simulink model "baopoco"  (Simulink 7.5)
settings: Solver=ode45  StartTime=0.0  StopTime=10.0  RelTol=1e-3
undefined symbols (not defined in the file or in its folder): quant
libraries: xbsIndex_r4 x4199, simulink x56, xps_library x32, dspsigops x24, casper_library_misc x9, casper_library_sources x4
(part 1 of 46)
settings Model: Creator=zaki  LastModifiedBy=zaki
settings DebuggingCC: StrictBusMsg=Warning

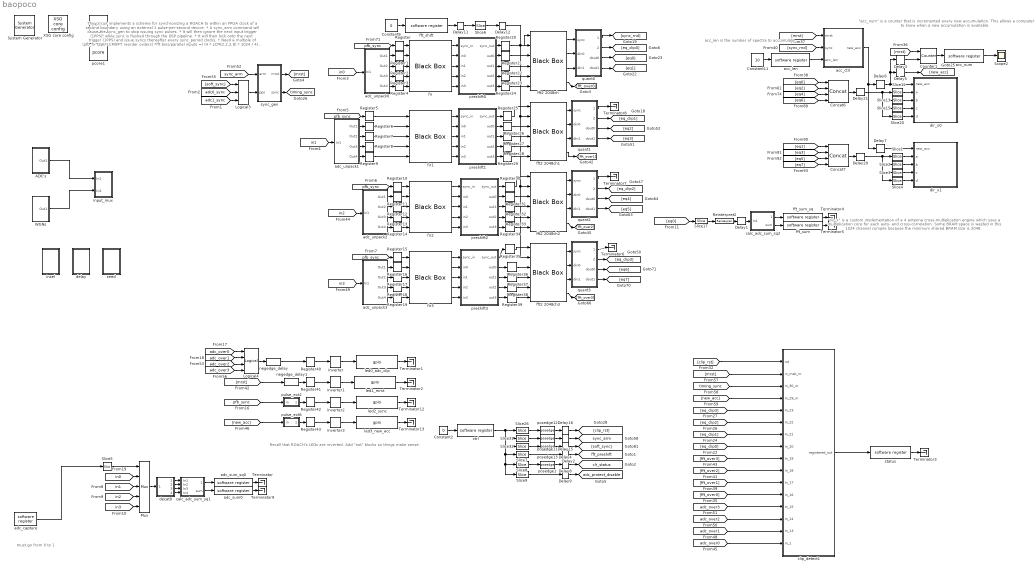
== system baopoco ==
  note: acc_len is the number of
      | spectra to accumulate.
  note: "acc_num" is a counter that is
      | incremented every new accumulation.
      | This allows a computer to know when
      | a new accumulation is available.
  note: "dir_x" is a custom implementation
      | of a 4 antenna cross-multiplication
      | engine which uses a multiplication
      | core for each auto- and cross-correlation.
      | Some BRAM space is wasted in this 1024
      | channel compile because the minimum shared
      | BRAM size is 2048.
  note: Recall that ROACH's LEDs are inverted.
      | Add "not" blocks so things make sense.
  note: This circuit implements a scheme for synchronizing a ROACH
      | to within an FPGA clock of a second boundary using an external 1 pulse-per-second device.
      |
      | * A sync_arm command will cause the sync_gen to stop issuing sync pulses.
      | * It will then ignore the next input trigger (1PPS), while sync is flushed through the DSP pipeline.
      | * It will then lock onto the next trigger (1PPS) and issue syncs thereafter every sync_period clocks.
      | * Need a multiple of (pfb_fir taps*LCM(FFT reorder orders) *fft bins/parallel inputs =)
      | (4 * LCM(2,2,2,8) * 1024 / 4) = 8192) for sync_period for simulation
      | * Trigger off either ADC's 1PPS inputs
  note: must go from 0 to 1
  " System Generator" : xbsIndex_r4/ System Generator  Tag=genX  SystemSampleTime=-1  xilinxfamily=virtex5  part=xc5vsx95t  speed=-1  package=ff1136  synthesis_tool=XST  clock_wrapper=Clock Enables  directory=./baopoco/sysgen  proj_type=Project Navigator  Synth_file=XST Defaults  Impl_file=ISE Defaults  testbench=off  simulink_period=1  sysclk_period=6.6667  dcm_input_clock_period=100  incr_netlist=off  trim_vbits=Everywhere in SubSystem  dbl_ovrd=According to Block Masks  core_generation=According to Block Masks  run_coregen=off  deprecated_control=off  eval_field=0  block_type=sysgen
      settings: block_version=11.4
  ADC's : Subsystem
  Concat6 : xbsIndex_r4/Concat  num_inputs=4  dbl_ovrd=off  block_type=concat
      settings: block_version=10.1.3
  Concat7 : xbsIndex_r4/Concat  num_inputs=4  dbl_ovrd=off  block_type=concat
      settings: block_version=10.1.3
  Constant11 : Constant  Value=10  OutDataType=sfix(16)  OutScaling=2^0
  Constant2 : Constant  Value=0  OutDataType=sfix(16)  OutScaling=2^0
  Constant9 : Constant  Value=0  OutDataType=sfix(16)  OutScaling=2^0
  Counter1 : xbsIndex_r4/Counter  cnt_type=Free Running  cnt_to=Inf  operation=Up  start_count=0  cnt_by_val=1  arith_type=Unsigned  n_bits=32  bin_pt=0  load_pin=off  rst=on  en=on  explicit_period=off  period=1  dbl_ovrd=off  use_behavioral_HDL=off  implementation=Fabric  use_rpm=on  block_type=counter
      settings: xl_area=[0, 0, 0, 0, 0, 0, 0]
  Delay1 : xbsIndex_r4/Delay  rst=off  infoeditControl=Selection of Reset will increase slice count due to use of real FFs and instead of SRLs  en=off  latency=2  dbl_ovrd=off  reg_retiming=on  block_type=delay
      settings: xl_area=[0, 0, 0, 0, 0, 0, 0]
  Delay{2,4..9,11..12,15..16,20..21} (13 alike) : xbsIndex_r4/Delay  rst=off  infoeditControl=Selection of Reset will increase slice count due to use of real FFs and instead of SRLs  en=off  latency=1  dbl_ovrd=off  reg_retiming=on  block_type=delay
      Delay20 settings: xl_area=[0, 0, 0, 0, 0, 0, 0]
      Delay21 settings: xl_area=[0, 0, 0, 0, 0, 0, 0]
      Delay5 settings: xl_area=[0, 0, 0, 0, 0, 0, 0]
      Delay6 settings: xl_area=[0, 0, 0, 0, 0, 0, 0]
      Delay7 settings: xl_area=[0, 0, 0, 0, 0, 0, 0]
  Delay3 : xbsIndex_r4/Delay  rst=off  infoeditControl=Selection of Reset will increase slice count due to use of real FFs and instead of SRLs  en=off  latency=2  dbl_ovrd=off  reg_retiming=on  block_type=delay
      settings: xl_area=[0, 0, 0, 0, 0, 0, 0]
  Inverter{,1..3} (4 alike) : xbsIndex_r4/Inverter  en=off  latency=2  dbl_ovrd=off  block_type=inv
  Logical3 : xbsIndex_r4/Logical  logical_function=OR  inputs=3  en=off  latency=0  precision=Full  arith_type=Unsigned  n_bits=16  bin_pt=0  align_bp=on  dbl_ovrd=off  block_type=logical
  Logical4 : xbsIndex_r4/Logical  logical_function=OR  inputs=4  en=off  latency=0  precision=Full  arith_type=Unsigned  n_bits=8  bin_pt=2  align_bp=on  dbl_ovrd=off  block_type=logical
      settings: xl_area=[0, 0, 0, 0, 0, 0, 0]
  Mux : xbsIndex_r4/Mux  inputs=4  en=off  latency=2  precision=Full  arith_type=Unsigned  n_bits=16  bin_pt=14  quantization=Truncate  overflow=Wrap  dbl_ovrd=off  block_type=mux
  Register{,1..43} (44 alike) : xbsIndex_r4/Register  init=0  rst=off  en=off  dbl_ovrd=off  block_type=register
  Reinterpret2 : xbsIndex_r4/Reinterpret  force_arith_type=on  arith_type="Signed  (2's comp)"  force_bin_pt=on  bin_pt=0  block_type=reinterpret
      settings: infoedit="Changes signal type without altering the binary representation.   You can changed the signal between signed and unsigned, and relocate the binary point.<br><br>Hardware notes: In hardware this block costs nothing.<br><br>Example:  Suppose the input is 6 bits wide, signed, with 2 fractional bits, and the output is forced to unsigned with 0 fractional bits.  Then an input of -2.0 (1110.00 in binary 2's complement) becomes an output of 56 (111000 in binary)."  block_version=10.1.3
  Scope2 : Scope
      settings: YMin=3  YMax=3
  Slice1 : xbsIndex_r4/Slice  nbits=8  boolean_output=off  mode=Lower Bit Location + Width  bit1=0  base1=MSB of Input  bit0=24  base0=LSB of Input  dbl_ovrd=off  block_type=slice
      settings: block_version=10.1.3
  Slice12 : xbsIndex_r4/Slice  nbits=8  boolean_output=off  mode=Lower Bit Location + Width  bit1=0  base1=MSB of Input  bit0=24  base0=LSB of Input  dbl_ovrd=off  block_type=slice
      settings: block_version=10.1.3
  Slice13 : xbsIndex_r4/Slice  nbits=8  boolean_output=off  mode=Lower Bit Location + Width  bit1=0  base1=MSB of Input  bit0=16  base0=LSB of Input  dbl_ovrd=off  block_type=slice
      settings: block_version=10.1.3
  Slice15 : xbsIndex_r4/Slice  nbits=8  boolean_output=off  mode=Lower Bit Location + Width  bit1=0  base1=MSB of Input  bit0=8  base0=LSB of Input  dbl_ovrd=off  block_type=slice
      settings: block_version=10.1.3
  Slice17 : xbsIndex_r4/Slice  nbits=4  boolean_output=off  mode=Lower Bit Location + Width  bit1=0  base1=MSB of Input  bit0=4  base0=LSB of Input  dbl_ovrd=off  block_type=slice
      settings: block_version=10.1.3
  Slice2 : xbsIndex_r4/Slice  nbits=8  boolean_output=off  mode=Lower Bit Location + Width  bit1=0  base1=MSB of Input  bit0=16  base0=LSB of Input  dbl_ovrd=off  block_type=slice
      settings: block_version=10.1.3
  Slice20 : xbsIndex_r4/Slice  nbits=8  boolean_output=off  mode=Lower Bit Location + Width  bit1=0  base1=MSB of Input  bit0=0  base0=LSB of Input  dbl_ovrd=off  block_type=slice
      settings: block_version=10.1.3
  Slice26 : xbsIndex_r4/Slice  nbits=1  boolean_output=on  mode=Lower Bit Location + Width  bit1=0  base1=MSB of Input  bit0=16  base0=LSB of Input  dbl_ovrd=off  block_type=slice
      settings: infoedit="Extracts a given range of bits from each input sample and presents it at the output.  The output type is ordinarily unsigned with binary point at zero, but can be Boolean when the slice is one bit wide.<P><P>Hardware notes: In hardware this block costs nothing."  block_version=10.1.3
  Slice3 : xbsIndex_r4/Slice  nbits=8  boolean_output=off  mode=Lower Bit Location + Width  bit1=0  base1=MSB of Input  bit0=8  base0=LSB of Input  dbl_ovrd=off  block_type=slice
      settings: block_version=10.1.3
  Slice31 : xbsIndex_r4/Slice  nbits=1  boolean_output=on  mode=Lower Bit Location + Width  bit1=0  base1=MSB of Input  bit0=17  base0=LSB of Input  dbl_ovrd=off  block_type=slice
      settings: infoedit="Extracts a given range of bits from each input sample and presents it at the output.  The output type is ordinarily unsigned with binary point at zero, but can be Boolean when the slice is one bit wide.<P><P>Hardware notes: In hardware this block costs nothing."  block_version=10.1.3
  Slice32 : xbsIndex_r4/Slice  nbits=1  boolean_output=on  mode=Lower Bit Location + Width  bit1=0  base1=MSB of Input  bit0=18  base0=LSB of Input  dbl_ovrd=off  block_type=slice
      settings: infoedit="Extracts a given range of bits from each input sample and presents it at the output.  The output type is ordinarily unsigned with binary point at zero, but can be Boolean when the slice is one bit wide.<P><P>Hardware notes: In hardware this block costs nothing."  block_version=10.1.3
  Slice4 : xbsIndex_r4/Slice  nbits=8  boolean_output=off  mode=Lower Bit Location + Width  bit1=0  base1=MSB of Input  bit0=0  base0=LSB of Input  dbl_ovrd=off  block_type=slice
      settings: block_version=10.1.3
  Slice5 : xbsIndex_r4/Slice  nbits=2  boolean_output=off  mode=Upper Bit Location + Width  bit1=0  base1=MSB of Input  bit0=0  base0=LSB of Input  dbl_ovrd=off  block_type=slice
  Slice6 : xbsIndex_r4/Slice  nbits=13  boolean_output=off  mode=Lower Bit Location + Width  bit1=0  base1=MSB of Input  bit0=0  base0=LSB of Input  dbl_ovrd=off  block_type=slice
      settings: infoedit="Extracts a given range of bits from each input sample and presents it at the output.  The output type is ordinarily unsigned with binary point at zero, but can be Boolean when the slice is one bit wide.<P><P>Hardware notes: In hardware this block costs nothing."  block_version=10.1.3
  Slice7 : xbsIndex_r4/Slice  nbits=2  boolean_output=off  mode=Lower Bit Location + Width  bit1=0  base1=MSB of Input  bit0=0  base0=LSB of Input  dbl_ovrd=off  block_type=slice
      settings: block_version=10.1.3
  Slice8 : xbsIndex_r4/Slice  nbits=1  boolean_output=on  mode=Lower Bit Location + Width  bit1=0  base1=MSB of Input  bit0=2  base0=LSB of Input  dbl_ovrd=off  block_type=slice
      settings: block_version=10.1.3
  Slice9 : xbsIndex_r4/Slice  nbits=1  boolean_output=on  mode=Lower Bit Location + Width  bit1=0  base1=MSB of Input  bit0=3  base0=LSB of Input  dbl_ovrd=off  block_type=slice
      settings: block_version=10.1.3
  Terminator{,1..9,12..13} (12 alike) : Terminator
  WGNs : Subsystem
  XSG core config : xps_library/XSG core config  Tag=xps:xsg  SystemSampleTime=-1  hw_sys=ROACH:sx95t  ibob_linux=off  clk_src=adc0_clk  iBOB_clk_src=sys_clk  BEE2_ctrl_clk_src=sys_clk  BEE2_usr_clk_src=sys_clk  ROACH_clk_src=adc0_clk  ROACH2_clk_src=adc0_clk  CORR_clk_src=sys_clk  gpio_clk_io_group=iBOB:sma  gpio_clk_bit_index=0  clk_rate=150  sample_period=1  synthesis_tool=XST  mpc_type=powerpc440_ext
  acc_ctrl : Subsystem  mask{chan_bits=9}
      mask prompts: chan_bits "Vector length (2^?)"
  acc_len : xps_library/software register  Tag=xps:sw_reg  SystemSampleTime=-1  io_dir=From Processor  arith_type=Unsigned  bitwidth=32  bin_pt=0  sample_period=1  gw_name=baopoco_acc_len_user_data_out
      link override baopoco_acc_len_user_data_out: arith_type=Unsigned  n_bits=32  bin_pt=0  period=1
  acc_num : xps_library/software register  Tag=xps:sw_reg  SystemSampleTime=-1  io_dir=To Processor  arith_type=Unsigned  bitwidth=32  bin_pt=0  sample_period=1  gw_name=baopoco_acc_num_user_data_in
      link override convert: arith_type=Unsigned  n_bits=32  bin_pt=0
  adc_capture : xps_library/software register  Tag=xps:sw_reg  SystemSampleTime=-1  io_dir=From Processor  arith_type=Unsigned  bitwidth=32  bin_pt=0  sample_period=1  gw_name=baopoco_adc_capture_user_data_out
      link override baopoco_adc_capture_user_data_out: arith_type=Unsigned  n_bits=32  bin_pt=0  period=1
  adc_sum0 : xps_library/software register  Tag=xps:sw_reg  SystemSampleTime=-1  io_dir=To Processor  arith_type=Unsigned  bitwidth=32  bin_pt=0  sample_period=1  gw_name=baopoco_adc_sum0_user_data_in
      link override convert: arith_type=Unsigned  n_bits=32  bin_pt=0
  adc_sum_sq0 : xps_library/software register  Tag=xps:sw_reg  SystemSampleTime=-1  io_dir=To Processor  arith_type=Unsigned  bitwidth=32  bin_pt=0  sample_period=1  gw_name=baopoco_adc_sum_sq0_user_data_in
      link override convert: arith_type=Unsigned  n_bits=32  bin_pt=0
  adc_unpack0 : Subsystem
  adc_unpack1 : Subsystem
  adc_unpack2 : Subsystem
  adc_unpack3 : Subsystem
  calc_adc_sum_sq1 : Subsystem
  calc_adc_sum_sq2 : Subsystem
  clip_detect1 : Subsystem
  ctrl : xps_library/software register  Tag=xps:sw_reg  SystemSampleTime=-1  io_dir=From Processor  arith_type=Unsigned  bitwidth=32  bin_pt=0  sample_period=1  gw_name=baopoco_ctrl_user_data_out
      link override baopoco_ctrl_user_data_out: arith_type=Unsigned  n_bits=32  bin_pt=0  period=1
  decat0 : Subsystem
  delay : Subsystem
      settings: AttributesFormatString=|
      | Addr 8195
      | 0x2003
  dir_x0 : Subsystem  mask{n_chans=512}
      mask prompts: n_chans "Number of Channels"
  dir_x1 : Subsystem  mask{n_chans=512}
      mask prompts: n_chans "Number of Channels"
  fft2 2048ch{,1..3} (4 alike) : xbsIndex_r4/Black Box  init_code=fft_wideband_real_core2_config  sim_method=Inactive  verbose=off  block_type=blackbox2
  fft_shift : xps_library/software register  Tag=xps:sw_reg  SystemSampleTime=-1  io_dir=From Processor  arith_type=Unsigned  bitwidth=32  bin_pt=0  sample_period=1  gw_name=baopoco_fft_shift_user_data_out
      link override baopoco_fft_shift_user_data_out: arith_type=Unsigned  n_bits=32  bin_pt=0  period=1
  fft_sum : xps_library/software register  Tag=xps:sw_reg  SystemSampleTime=-1  io_dir=To Processor  arith_type=Unsigned  bitwidth=32  bin_pt=0  sample_period=1  gw_name=baopoco_fft_sum_user_data_in
      link override convert: arith_type=Unsigned  n_bits=32  bin_pt=0
  fft_sum_sq : xps_library/software register  Tag=xps:sw_reg  SystemSampleTime=-1  io_dir=To Processor  arith_type=Unsigned  bitwidth=32  bin_pt=0  sample_period=1  gw_name=baopoco_fft_sum_sq_user_data_in
      link override convert: arith_type=Unsigned  n_bits=32  bin_pt=0
  fir{,1..3} (4 alike) : xbsIndex_r4/Black Box  init_code=fir_core_config  sim_method=Inactive  verbose=off  block_type=blackbox2
  input_mux : Subsystem
  insel : Subsystem
      settings: AttributesFormatString=|
      | Addr 8194
      | 0x2002
  led0_adc_clip : xps_library/gpio  Tag=xps:gpio  SystemSampleTime=-1  io_group=ROACH:led  io_dir=out  arith_type=Boolean  bitwidth=1  bin_pt=0  bit_index=0  sample_period=1  use_single_ended=off  use_ddr=off  reg_iob=on  reg_clk_phase=0  termination=None
      link override convert: arith_type=Boolean  n_bits=1  bin_pt=0
  led1_mrst : xps_library/gpio  Tag=xps:gpio  SystemSampleTime=-1  io_group=ROACH:led  io_dir=out  arith_type=Boolean  bitwidth=1  bin_pt=0  bit_index=1  sample_period=1  use_single_ended=off  use_ddr=off  reg_iob=on  reg_clk_phase=0  termination=None
      link override convert: arith_type=Boolean  n_bits=1  bin_pt=0
  led2_sync : xps_library/gpio  Tag=xps:gpio  SystemSampleTime=-1  io_group=ROACH:led  io_dir=out  arith_type=Boolean  bitwidth=1  bin_pt=0  bit_index=2  sample_period=1  use_single_ended=off  use_ddr=off  reg_iob=on  reg_clk_phase=0  termination=None
      link override convert: arith_type=Boolean  n_bits=1  bin_pt=0
  led3_new_acc : xps_library/gpio  Tag=xps:gpio  SystemSampleTime=-1  io_group=ROACH:led  io_dir=out  arith_type=Unsigned  bitwidth=1  bin_pt=0  bit_index=3  sample_period=1  use_single_ended=off  use_ddr=off  reg_iob=on  reg_clk_phase=0  termination=None
      link override convert: arith_type=Unsigned  n_bits=1  bin_pt=0
  negedge_delay : casper_library_misc/negedge_delay  SystemSampleTime=-1  pulse_len=2^24
  negedge_delay1 : casper_library_misc/negedge_delay  SystemSampleTime=-1  pulse_len=2^24
  pcore : xps_library/pcore  Tag=xps:pcore  SystemSampleTime=-1  pcore_path=./fft_wideband_real_core2/fft_wideband_real_core2.ngc
  pcore1 : xps_library/pcore  Tag=xps:pcore  SystemSampleTime=-1  pcore_path=./fir_core/fir_core.ngc
  posedge{1,11..13} (4 alike) : casper_library_misc/posedge  Tag=Rising Edge Detector  SystemSampleTime=-1
  preshift0 : Subsystem
  preshift1 : Subsystem
  preshift2 : Subsystem
  preshift3 : Subsystem
  pulse_ext2 : Subsystem  mask{pulse_len=2^24}
      mask prompts: pulse_len "Length of Pulse"
      mask init:
      | bits = ceil(log2(pulse_len+1));
  pulse_ext6 : Subsystem  mask{pulse_len=2^24}
      mask prompts: pulse_len "Length of Pulse"
      mask init:
      | bits = ceil(log2(pulse_len+1));
  quant0 : Subsystem
  quant1 : Subsystem
  quant2 : Subsystem
  quant3 : Subsystem
  seed : Subsystem
      settings: AttributesFormatString=|
      | Addr 8196
      | 0x2004
  status : xps_library/software register  Tag=xps:sw_reg  SystemSampleTime=-1  io_dir=To Processor  arith_type=Unsigned  bitwidth=32  bin_pt=0  sample_period=1  gw_name=baopoco_status_user_data_in
      link override convert: arith_type=Unsigned  n_bits=32  bin_pt=0
  sync_gen : Subsystem  mask(sync_gen_hw){sync_period_bits=27}
      mask prompts: sync_period_bits "Size of sync generator counter (bits)"
      mask help:
      | This circuit implements a scheme for synchronizing a design to within an FPGA clock of an external 1 pulse-per-second signal.
      |
      | * An arm pulse will cause the sync_gen to stop issuing sync pulses.
      | * It will then ignore the next input trigger (1PPS), while sync is flushed through the DSP pipeline.
      | * It will then lock onto the next trigger (1PPS) and issue syncs thereafter every sync_period clocks.
      | * You will need a multiple of (pfb_fir taps*LCM(FFT reorder orders) *fft bins/parallel inputs). See the sync memo for further details.
  tag scope: adc_protect_disable=global, clr_status=global, fft_preshift=global, sync_arm=global, timing_sync=global
  wires:
  [adc0_sync] -> Logical3/2
  sync_gen/1(mrst) -> [mrst]
  sync_gen/2(sync) -> [timing_sync]
  Logical3/1 -> sync_gen/2(pps)
  [soft_sync] -> Logical3/1
  [adc1_sync] -> Logical3/3
  [sync_arm] -> sync_gen/1(arm)
  Constant2/1 -> ctrl/1
  ctrl/1 -> Slice31/1, Slice32/1, Slice7/1, Slice8/1, Slice9/1, Slice26/1
  Slice26/1 -> posedge12/1
  Slice31/1 -> posedge11/1
  Slice32/1 -> posedge13/1
  posedge11/1 -> Delay15/1
  posedge12/1 -> Delay16/1
  posedge13/1 -> Delay4/1
  Delay4/1 -> [soft_sync]
  Delay15/1 -> [sync_arm]
  Delay16/1 -> [clip_rst]
  Constant9/1 -> fft_shift/1
  fft_shift/1 -> Delay11/1
  fir/2 -> preshift0/2(in0)
  fir/3 -> preshift0/3(in1)
  fir/4 -> preshift0/4(in2)
  fir/5 -> preshift0/5(in3)
  preshift0/2(out0) -> Register21/1
  preshift0/3(out1) -> Register22/1
  preshift0/4(out2) -> Register23/1
  preshift0/5(out3) -> Register24/1
  Slice6/1 -> Delay12/1
  fir/1 -> preshift0/1(sync_in)
  preshift0/1(sync_out) -> Register20/1
  fft2 2048ch/4 -> [fft_over0]
  [in0] -> adc_unpack0/1(In1)
  fir1/2 -> preshift1/2(in0)
  fir1/3 -> preshift1/3(in1)
  fir1/4 -> preshift1/4(in2)
  fir1/5 -> preshift1/5(in3)
  preshift1/2(out0) -> Register26/1
  preshift1/3(out1) -> Register27/1
  preshift1/4(out2) -> Register28/1
  preshift1/5(out3) -> Register29/1
  fir1/1 -> preshift1/1(sync_in)
  preshift1/1(sync_out) -> Register25/1
  fft2 2048ch1/4 -> [fft_over1]
  fir2/2 -> preshift2/2(in0)
  fir2/3 -> preshift2/3(in1)
  fir2/4 -> preshift2/4(in2)
  fir2/5 -> preshift2/5(in3)
  preshift2/2(out0) -> Register31/1
  preshift2/3(out1) -> Register32/1
  preshift2/4(out2) -> Register33/1
  preshift2/5(out3) -> Register34/1
  fir2/1 -> preshift2/1(sync_in)
  preshift2/1(sync_out) -> Register30/1
  fir3/2 -> preshift3/2(in0)
  fir3/3 -> preshift3/3(in1)
  fir3/4 -> preshift3/4(in2)
  fir3/5 -> preshift3/5(in3)
  preshift3/2(out0) -> Register36/1
  preshift3/3(out1) -> Register37/1
  preshift3/4(out2) -> Register38/1
  preshift3/5(out3) -> Register39/1
  fir3/1 -> preshift3/1(sync_in)
  preshift3/1(sync_out) -> Register35/1
  quant0/1(sync_out) -> [sync_rnd]
  quant0/3(dout0) -> [eq0]
  quant0/4(dout1) -> [eq1]
  fft2 2048ch2/4 -> [fft_over2]
  fft2 2048ch3/4 -> [fft_over3]
  quant0/2(clip_out) -> [eq_clip0]
  fft2 2048ch/2 -> quant0/2(din0)
  fft2 2048ch/3 -> quant0/3(din1)
  fft2 2048ch/1 -> quant0/1(sync)
  quant1/1(sync_out) -> Terminator8/1
  quant1/3(dout0) -> [eq2]
  quant1/4(dout1) -> [eq3]
  quant1/2(clip_out) -> [eq_clip1]
  quant2/1(sync_out) -> Terminator7/1
  quant2/3(dout0) -> [eq4]
  quant2/2(clip_out) -> [eq_clip2]
  quant3/1(sync_out) -> Terminator6/1
  quant3/3(dout0) -> [eq6]
  quant3/4(dout1) -> [eq7]
  quant3/2(clip_out) -> [eq_clip3]
  [in1] -> adc_unpack1/1(In1)
  [in2] -> adc_unpack2/1(In1)
  [in3] -> adc_unpack3/1(In1)
  fft2 2048ch1/1 -> quant1/1(sync)
  fft2 2048ch1/2 -> quant1/2(din0)
  fft2 2048ch1/3 -> quant1/3(din1)
  fft2 2048ch2/1 -> quant2/1(sync)
  fft2 2048ch2/2 -> quant2/2(din0)
  fft2 2048ch2/3 -> quant2/3(din1)
  fft2 2048ch3/1 -> quant3/1(sync)
  fft2 2048ch3/2 -> quant3/2(din0)
  fft2 2048ch3/3 -> quant3/3(din1)
  quant2/4(dout1) -> [eq5]
  Constant11/1 -> acc_len/1
  acc_len/1 -> acc_ctrl/3(acc_len)
  Concat6/1 -> Delay21/1
  Slice12/1 -> dir_x0/2(a)
  Slice13/1 -> dir_x0/3(b)
  Slice15/1 -> dir_x0/4(c)
  Slice20/1 -> dir_x0/5(d)
  acc_ctrl/1(new_acc) -> Delay7/1, Delay6/1
  [mrst] -> acc_ctrl/1(mrst)
  [sync_rnd] -> acc_ctrl/2(sync)
  Delay6/1 -> Delay5/1, Delay3/1, dir_x0/1(new_acc)
  [eq0] -> Concat6/1
  [eq2] -> Concat6/2
  [eq4] -> Concat6/3
  [eq6] -> Concat6/4
  [eq1] -> Concat7/1
  [eq3] -> Concat7/2
  [eq5] -> Concat7/3
  [eq7] -> Concat7/4
  Delay20/1 -> Slice2/1, Slice3/1, Slice4/1, Slice1/1
  Concat7/1 -> Delay20/1
  Delay21/1 -> Slice20/1, Slice15/1, Slice13/1, Slice12/1
  Counter1/1 -> acc_num/1
  acc_num/1 -> Scope2/1
  [mrst] -> Counter1/1
  Delay3/1 -> Counter1/2
  Delay5/1 -> [new_acc]
  [clip_rst] -> clip_detect1/1(rst)
  [adc_over3] -> clip_detect1/13(In_15)
  [adc_over2] -> clip_detect1/14(In_14)
  [adc_over1] -> clip_detect1/15(In_13)
  [adc_over0] -> clip_detect1/16(In_1)
  [mrst] -> clip_detect1/2(in_msb_nr)
  clip_detect1/1(registered_out) -> status/1
  status/1 -> Terminator3/1
  pulse_ext2/1(out) -> Register42/1
  [pfb_sync] -> pulse_ext2/1(in)
  [mrst] -> negedge_delay1/1
  pulse_ext6/1(out) -> Register43/1
  [new_acc] -> pulse_ext6/1(in)
  led0_adc_clip/1 -> Terminator1/1
  led1_mrst/1 -> Terminator2/1
  led2_sync/1 -> Terminator12/1
  led3_new_acc/1 -> Terminator13/1
  Logical4/1 -> negedge_delay/1
  negedge_delay/1 -> Register40/1
  [adc_over0] -> Logical4/1
  [adc_over1] -> Logical4/2
  [adc_over2] -> Logical4/3
  [adc_over3] -> Logical4/4
  negedge_delay1/1 -> Register41/1
  Inverter/1 -> led0_adc_clip/1
  Inverter1/1 -> led1_mrst/1
  Inverter2/1 -> led2_sync/1
  Inverter3/1 -> led3_new_acc/1
  calc_adc_sum_sq1/1(sum_sq) -> adc_sum_sq0/1
  decat0/1(Out1) -> calc_adc_sum_sq1/1(In1)
  decat0/2(Out2) -> calc_adc_sum_sq1/2(In2)
  decat0/3(Out3) -> calc_adc_sum_sq1/3(In3)
  decat0/4(Out4) -> calc_adc_sum_sq1/4(In4)
  adc_sum_sq0/1 -> Terminator/1
  adc_sum0/1 -> Terminator9/1
  calc_adc_sum_sq1/2(sum) -> adc_sum0/1
  WGNs/1(Out1) -> input_mux/2(In2)
  ADC's/1(Out1) -> input_mux/1(In1)
  adc_capture/1 -> Slice5/1
  Slice1/1 -> dir_x1/2(a)
  Slice2/1 -> dir_x1/3(b)
  Slice3/1 -> dir_x1/4(c)
  Slice4/1 -> dir_x1/5(d)
  Delay7/1 -> dir_x1/1(new_acc)
  [pfb_sync] -> Register/1
  Register/1 -> fir/1
  adc_unpack0/1(Out1) -> Register1/1
  Register1/1 -> fir/2
  adc_unpack0/2(Out2) -> Register2/1
  Register2/1 -> fir/3
  adc_unpack0/3(Out3) -> Register3/1
  Register3/1 -> fir/4
  adc_unpack0/4(Out4) -> Register4/1
  Register4/1 -> fir/5
  [pfb_sync] -> Register5/1
  Register5/1 -> fir1/1
  adc_unpack1/1(Out1) -> Register6/1
  Register6/1 -> fir1/2
  adc_unpack1/2(Out2) -> Register7/1
  Register7/1 -> fir1/3
  adc_unpack1/3(Out3) -> Register8/1
  Register8/1 -> fir1/4
  adc_unpack1/4(Out4) -> Register9/1
  Register9/1 -> fir1/5
  [pfb_sync] -> Register10/1
  Register10/1 -> fir2/1
  adc_unpack2/1(Out1) -> Register11/1
  Register11/1 -> fir2/2
  adc_unpack2/2(Out2) -> Register12/1
  Register12/1 -> fir2/3
  adc_unpack2/3(Out3) -> Register13/1
  Register13/1 -> fir2/4
  adc_unpack2/4(Out4) -> Register14/1
  Register14/1 -> fir2/5
  [pfb_sync] -> Register15/1
  Register15/1 -> fir3/1
  adc_unpack3/1(Out1) -> Register16/1
  Register16/1 -> fir3/2
  adc_unpack3/2(Out2) -> Register17/1
  Register17/1 -> fir3/3
  adc_unpack3/3(Out3) -> Register18/1
  Register18/1 -> fir3/4
  adc_unpack3/4(Out4) -> Register19/1
  Register19/1 -> fir3/5
  Delay11/1 -> Slice6/1
  Delay12/1 -> fft2 2048ch2/2, fft2 2048ch3/2, fft2 2048ch1/2, fft2 2048ch/2
  Register20/1 -> fft2 2048ch/1
  Register21/1 -> fft2 2048ch/3
  Register22/1 -> fft2 2048ch/4
  Register23/1 -> fft2 2048ch/5
  Register24/1 -> fft2 2048ch/6
  Register25/1 -> fft2 2048ch1/1
  Register26/1 -> fft2 2048ch1/3
  Register27/1 -> fft2 2048ch1/4
  Register28/1 -> fft2 2048ch1/5
  Register29/1 -> fft2 2048ch1/6
  Register30/1 -> fft2 2048ch2/1
  Register31/1 -> fft2 2048ch2/3
  Register32/1 -> fft2 2048ch2/4
  Register33/1 -> fft2 2048ch2/5
  Register34/1 -> fft2 2048ch2/6
  Register35/1 -> fft2 2048ch3/1
  Register36/1 -> fft2 2048ch3/3
  Register37/1 -> fft2 2048ch3/4
  Register38/1 -> fft2 2048ch3/5
  Register39/1 -> fft2 2048ch3/6
  Mux/1 -> decat0/1(In1)
  [in0] -> Mux/2
  [in1] -> Mux/3
  [in3] -> Mux/5
  [in2] -> Mux/4
  Slice5/1 -> Mux/1
  [fft_over0] -> clip_detect1/12(In_16)
  [fft_over1] -> clip_detect1/11(In_17)
  [fft_over2] -> clip_detect1/10(In_18)
  [fft_over3] -> clip_detect1/9(In_19)
  [eq_clip0] -> clip_detect1/8(In_20)
  [eq_clip1] -> clip_detect1/7(In_21)
  [eq_clip2] -> clip_detect1/6(In_22)
  [eq_clip3] -> clip_detect1/5(In_23)
  [timing_sync] -> clip_detect1/3(In_30_nr)
  [new_acc] -> clip_detect1/4(In_29_nr)
  Register40/1 -> Inverter/1
  Register41/1 -> Inverter1/1
  Register42/1 -> Inverter2/1
  Register43/1 -> Inverter3/1
  calc_adc_sum_sq2/1(sum_sq) -> fft_sum_sq/1
  fft_sum_sq/1 -> Terminator4/1
  fft_sum/1 -> Terminator5/1
  calc_adc_sum_sq2/2(sum) -> fft_sum/1
  Slice17/1 -> Reinterpret2/1
  Reinterpret2/1 -> Delay1/1
  [eq0] -> Slice17/1
  Delay1/1 -> calc_adc_sum_sq2/1(In1)
  Slice7/1 -> Delay2/1
  Delay2/1 -> [fft_preshift]
  Slice8/1 -> posedge1/1
  posedge1/1 -> Delay8/1
  Delay8/1 -> [clr_status]
  Slice9/1 -> Delay9/1
  Delay9/1 -> [adc_protect_disable]

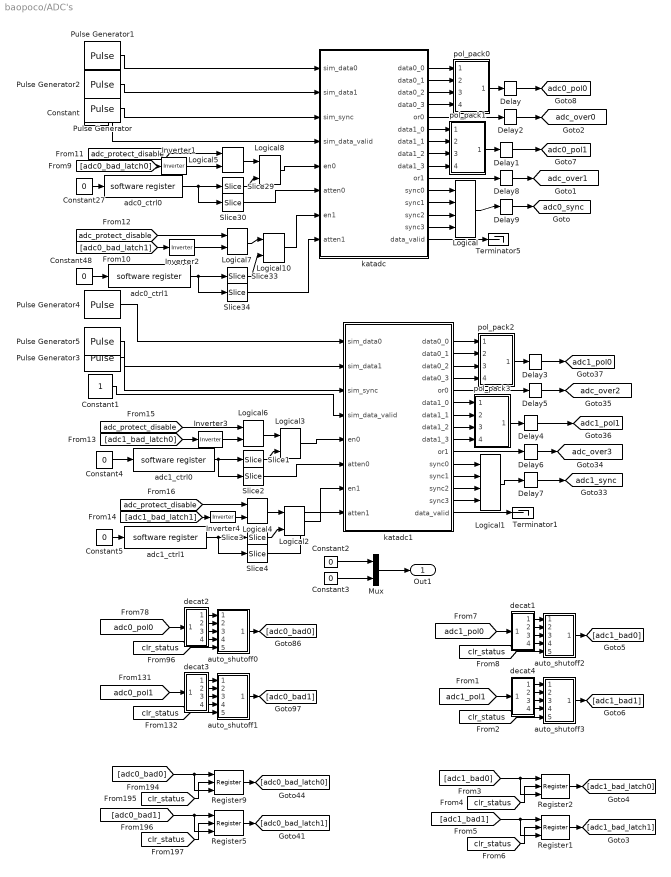
== system baopoco/ADC's ==
  Constant : Constant  OutDataType=sfix(16)  OutScaling=2^0  SampleTime=1/4
  Constant1 : Constant  OutDataType=sfix(16)  OutScaling=2^0  SampleTime=1/4
  Constant2 : Constant  Value=0
  Constant{4..5,27,48} (4 alike) : Constant  Value=0  OutDataType=sfix(16)  OutScaling=2^0
  Constant3 : Constant  Value=0
  Delay{,1..9} (10 alike) : xbsIndex_r4/Delay  rst=off  infoeditControl=Selection of Reset will increase slice count due to use of real FFs and instead of SRLs  en=off  latency=1  dbl_ovrd=off  reg_retiming=on  block_type=delay
  Inverter{1..4} (4 alike) : xbsIndex_r4/Inverter  en=off  latency=0  dbl_ovrd=off  block_type=inv
      settings: block_version=11.4
  Logical : xbsIndex_r4/Logical  logical_function=OR  inputs=4  en=off  latency=0  precision=Full  arith_type=Unsigned  n_bits=16  bin_pt=0  align_bp=on  dbl_ovrd=off  block_type=logical
  Logical1 : xbsIndex_r4/Logical  logical_function=OR  inputs=4  en=off  latency=0  precision=Full  arith_type=Unsigned  n_bits=16  bin_pt=0  align_bp=on  dbl_ovrd=off  block_type=logical
  Logical{2..3,8,10} (4 alike) : xbsIndex_r4/Logical  logical_function=AND  inputs=2  en=off  latency=0  precision=Full  arith_type=Unsigned  n_bits=16  bin_pt=0  align_bp=on  dbl_ovrd=off  block_type=logical
  Logical{4..7} (4 alike) : xbsIndex_r4/Logical  logical_function=OR  inputs=2  en=off  latency=0  precision=Full  arith_type=Unsigned  n_bits=16  bin_pt=0  align_bp=on  dbl_ovrd=off  block_type=logical
  Mux : Mux  Inputs=2
  Pulse Generator : DiscretePulseGenerator  Amplitude=1  Period=2^8  PulseWidth=1  PhaseDelay=11  SampleTime=1/4
  Pulse Generator1 : DiscretePulseGenerator  Amplitude=0.75  Period=2^10  PulseWidth=1  PhaseDelay=9+13+2^8  SampleTime=1/4
  Pulse Generator2 : DiscretePulseGenerator  Amplitude=0.5  Period=2^10  PulseWidth=1  PhaseDelay=9+14+2^8  SampleTime=1/4
  Pulse Generator3 : DiscretePulseGenerator  Amplitude=1  Period=2^8  PulseWidth=1  PhaseDelay=11  SampleTime=1/4
  Pulse Generator4 : DiscretePulseGenerator  Amplitude=0.75  Period=2^10  PulseWidth=1  PhaseDelay=9+13+2^8  SampleTime=1/4
  Pulse Generator5 : DiscretePulseGenerator  Amplitude=0.5  Period=2^10  PulseWidth=1  PhaseDelay=9+14+2^8  SampleTime=1/4
  Register{1..2,5,9} (4 alike) : xbsIndex_r4/Register  init=0  rst=on  en=on  dbl_ovrd=off  block_type=register
  Slice{1,3,29,33} (4 alike) : xbsIndex_r4/Slice  nbits=1  boolean_output=on  mode=Upper Bit Location + Width  bit1=0  base1=MSB of Input  bit0=0  base0=LSB of Input  dbl_ovrd=off  block_type=slice
  Slice{2,4,30,34} (4 alike) : xbsIndex_r4/Slice  nbits=6  boolean_output=off  mode=Lower Bit Location + Width  bit1=0  base1=MSB of Input  bit0=0  base0=LSB of Input  dbl_ovrd=off  block_type=slice
  Terminator1 : Terminator
  Terminator5 : Terminator
  adc0_ctrl0 : xps_library/software register  Tag=xps:sw_reg  SystemSampleTime=-1  io_dir=From Processor  arith_type=Unsigned  bitwidth=32  bin_pt=0  sample_period=1  gw_name=baopoco_ADC_s_adc0_ctrl0_user_data_out
  adc0_ctrl1 : xps_library/software register  Tag=xps:sw_reg  SystemSampleTime=-1  io_dir=From Processor  arith_type=Unsigned  bitwidth=32  bin_pt=0  sample_period=1  gw_name=baopoco_ADC_s_adc0_ctrl1_user_data_out
  adc1_ctrl0 : xps_library/software register  Tag=xps:sw_reg  SystemSampleTime=-1  io_dir=From Processor  arith_type=Unsigned  bitwidth=32  bin_pt=0  sample_period=1  gw_name=baopoco_ADC_s_adc1_ctrl0_user_data_out
  adc1_ctrl1 : xps_library/software register  Tag=xps:sw_reg  SystemSampleTime=-1  io_dir=From Processor  arith_type=Unsigned  bitwidth=32  bin_pt=0  sample_period=1  gw_name=baopoco_ADC_s_adc1_ctrl1_user_data_out
  auto_shutoff0 : Subsystem  mask{bits_in=8, bin_pt_in=7, clk_freq=200, acc_time=20, max_power=1/sqrt(2)}
      mask prompts: bits_in "Input bits"; bin_pt_in "Input binary point"; clk_freq "Clock frequency (MHz)"; acc_time "Window (ms)"; max_power "Max power allowed"
  auto_shutoff1 : Subsystem  mask{bits_in=8, bin_pt_in=7, clk_freq=200, acc_time=20, max_power=1/sqrt(2)}
      mask prompts: bits_in "Input bits"; bin_pt_in "Input binary point"; clk_freq "Clock frequency (MHz)"; acc_time "Window (ms)"; max_power "Max power allowed"
  auto_shutoff2 : Subsystem  mask{bits_in=8, bin_pt_in=7, clk_freq=200, acc_time=20, max_power=1/sqrt(2)}
      mask prompts: bits_in "Input bits"; bin_pt_in "Input binary point"; clk_freq "Clock frequency (MHz)"; acc_time "Window (ms)"; max_power "Max power allowed"
  auto_shutoff3 : Subsystem  mask{bits_in=8, bin_pt_in=7, clk_freq=200, acc_time=20, max_power=1/sqrt(2)}
      mask prompts: bits_in "Input bits"; bin_pt_in "Input binary point"; clk_freq "Clock frequency (MHz)"; acc_time "Window (ms)"; max_power "Max power allowed"
  decat1 : Subsystem
  decat2 : Subsystem
  decat3 : Subsystem
  decat4 : Subsystem
  katadc : Subsystem  Tag=xps:katadc  AncestorBlock=xps_library/katadc  mask(katadc){adc_brd=adc0, adc_interleave=off, bypass_auto=off, en_gain=on, adc_clk_rate=600, sample_period=1}
      mask prompts: adc_brd "ADC board"; adc_interleave "ADC interleave mode"; bypass_auto "Bypass auto config"; en_gain "Enable gain"; adc_clk_rate "ADC sampling rate (MHz)"; sample_period "Sample period"
      mask init:
      | name = gcb;
      | katadc_init(gcb, ...
      |     'name', name, ...
      |     'adc_brd', adc_brd, ...
      |     'adc_interleave', adc_interleave, ...
      |     'bypass_auto', bypass_auto, ...
      |     'en_gain', en_gain, ...
      |     'adc_clk_rate', adc_clk_rate, ...
      |     'sample_period', sample_period);
      mask StyleString: popup(adc0|adc1),checkbox,checkbox,checkbox,edit,edit
  katadc1 : Subsystem  Tag=xps:katadc  AncestorBlock=xps_library/katadc  mask(katadc){adc_brd=adc1, adc_interleave=off, bypass_auto=off, en_gain=on, adc_clk_rate=600, sample_period=1}
      mask prompts: adc_brd "ADC board"; adc_interleave "ADC interleave mode"; bypass_auto "Bypass auto config"; en_gain "Enable gain"; adc_clk_rate "ADC sampling rate (MHz)"; sample_period "Sample period"
      mask init: same as baopoco/ADC's/katadc
      mask StyleString: popup(adc0|adc1),checkbox,checkbox,checkbox,edit,edit
  pol_pack0 : Subsystem
  pol_pack1 : Subsystem
  pol_pack2 : Subsystem
  pol_pack3 : Subsystem
  Out1 : Outport  OutDataType=sfix(16)  OutScaling=2^0
  tag scope: adc0_pol0=global, adc0_pol1=global, adc0_sync=global, adc1_pol0=global, adc1_pol1=global, adc1_sync=global, adc_over0=global, adc_over1=global, adc_over2=global, adc_over3=global
  wires:
  Delay/1 -> [adc0_pol0]
  Delay1/1 -> [adc0_pol1]
  Delay2/1 -> [adc_over0]
  Delay8/1 -> [adc_over1]
  Delay9/1 -> [adc0_sync]
  katadc/4(data0_3) -> pol_pack0/4(In4)
  katadc/3(data0_2) -> pol_pack0/3(In3)
  katadc/2(data0_1) -> pol_pack0/2(In2)
  katadc/1(data0_0) -> pol_pack0/1(In1)
  katadc/10(or1) -> Delay8/1
  pol_pack0/1(Out1) -> Delay/1
  pol_pack1/1(Out1) -> Delay1/1
  Logical/1 -> Delay9/1
  Constant/1 -> katadc/4(sim_data_valid)
  Pulse Generator2/1 -> katadc/2(sim_data1)
  Pulse Generator1/1 -> katadc/1(sim_data0)
  Pulse Generator/1 -> katadc/3(sim_sync)
  Constant3/1 -> Mux/2
  Constant2/1 -> Mux/1
  Mux/1 -> Out1/1
  katadc/5(or0) -> Delay2/1
  katadc/6(data1_0) -> pol_pack1/1(In1)
  katadc/7(data1_1) -> pol_pack1/2(In2)
  katadc/8(data1_2) -> pol_pack1/3(In3)
  katadc/9(data1_3) -> pol_pack1/4(In4)
  katadc/11(sync0) -> Logical/1
  katadc/12(sync1) -> Logical/2
  katadc/13(sync2) -> Logical/3
  katadc/14(sync3) -> Logical/4
  katadc/15(data_valid) -> Terminator5/1
  Delay3/1 -> [adc1_pol0]
  Delay7/1 -> [adc1_sync]
  katadc1/4(data0_3) -> pol_pack2/4(In4)
  katadc1/3(data0_2) -> pol_pack2/3(In3)
  katadc1/2(data0_1) -> pol_pack2/2(In2)
  katadc1/1(data0_0) -> pol_pack2/1(In1)
  katadc1/10(or1) -> Delay6/1
  pol_pack2/1(Out1) -> Delay3/1
  pol_pack3/1(Out1) -> Delay4/1
  Logical1/1 -> Delay7/1
  katadc1/5(or0) -> Delay5/1
  katadc1/6(data1_0) -> pol_pack3/1(In1)
  katadc1/7(data1_1) -> pol_pack3/2(In2)
  katadc1/8(data1_2) -> pol_pack3/3(In3)
  katadc1/9(data1_3) -> pol_pack3/4(In4)
  katadc1/11(sync0) -> Logical1/1
  katadc1/12(sync1) -> Logical1/2
  katadc1/13(sync2) -> Logical1/3
  katadc1/14(sync3) -> Logical1/4
  katadc1/15(data_valid) -> Terminator1/1
  Delay5/1 -> [adc_over2]
  Delay4/1 -> [adc1_pol1]
  Delay6/1 -> [adc_over3]
  Pulse Generator4/1 -> katadc1/1(sim_data0)
  Pulse Generator5/1 -> katadc1/2(sim_data1)
  Pulse Generator3/1 -> katadc1/3(sim_sync)
  Constant1/1 -> katadc1/4(sim_data_valid)
  Constant27/1 -> adc0_ctrl0/1
  adc0_ctrl0/1 -> Slice29/1, Slice30/1
  Slice29/1 -> Logical8/2
  Logical8/1 -> katadc/5(en0)
  Slice30/1 -> katadc/6(atten0)
  Constant48/1 -> adc0_ctrl1/1
  adc0_ctrl1/1 -> Slice34/1, Slice33/1
  Slice33/1 -> Logical10/2
  Logical10/1 -> katadc/7(en1)
  Slice34/1 -> katadc/8(atten1)
  Constant4/1 -> adc1_ctrl0/1
  adc1_ctrl0/1 -> Slice1/1, Slice2/1
  Slice1/1 -> Logical3/2
  Slice2/1 -> katadc1/6(atten0)
  Constant5/1 -> adc1_ctrl1/1
  adc1_ctrl1/1 -> Slice4/1, Slice3/1
  Slice3/1 -> Logical2/2
  Slice4/1 -> katadc1/8(atten1)
  Logical3/1 -> katadc1/5(en0)
  Logical2/1 -> katadc1/7(en1)
  [adc0_pol0] -> decat2/1(i1)
  decat2/1(o1) -> auto_shutoff0/1(In1)
  decat2/2(o2) -> auto_shutoff0/2(In2)
  decat2/3(o3) -> auto_shutoff0/3(In3)
  decat2/4(o4) -> auto_shutoff0/4(In4)
  [clr_status] -> auto_shutoff0/5(rst)
  auto_shutoff0/1(en) -> [adc0_bad0]
  [adc0_pol1] -> decat3/1(i1)
  decat3/1(o1) -> auto_shutoff1/1(In1)
  decat3/2(o2) -> auto_shutoff1/2(In2)
  decat3/3(o3) -> auto_shutoff1/3(In3)
  decat3/4(o4) -> auto_shutoff1/4(In4)
  [clr_status] -> auto_shutoff1/5(rst)
  auto_shutoff1/1(en) -> [adc0_bad1]
  Register9/1 -> [adc0_bad_latch0]
  [adc0_bad0] -> Register9/1, Register9/3
  [clr_status] -> Register9/2
  Register5/1 -> [adc0_bad_latch1]
  [adc0_bad1] -> Register5/3, Register5/1
  [clr_status] -> Register5/2
  [adc1_pol0] -> decat1/1(i1)
  decat1/1(o1) -> auto_shutoff2/1(In1)
  decat1/2(o2) -> auto_shutoff2/2(In2)
  decat1/3(o3) -> auto_shutoff2/3(In3)
  decat1/4(o4) -> auto_shutoff2/4(In4)
  [clr_status] -> auto_shutoff2/5(rst)
  auto_shutoff2/1(en) -> [adc1_bad0]
  [adc1_pol1] -> decat4/1(i1)
  decat4/1(o1) -> auto_shutoff3/1(In1)
  decat4/2(o2) -> auto_shutoff3/2(In2)
  decat4/3(o3) -> auto_shutoff3/3(In3)
  decat4/4(o4) -> auto_shutoff3/4(In4)
  [clr_status] -> auto_shutoff3/5(rst)
  auto_shutoff3/1(en) -> [adc1_bad1]
  Register2/1 -> [adc1_bad_latch0]
  [adc1_bad0] -> Register2/1, Register2/3
  [clr_status] -> Register2/2
  Register1/1 -> [adc1_bad_latch1]
  [adc1_bad1] -> Register1/3, Register1/1
  [clr_status] -> Register1/2
  [adc0_bad_latch0] -> Inverter1/1
  [adc0_bad_latch1] -> Inverter2/1
  [adc1_bad_latch1] -> Inverter4/1
  [adc1_bad_latch0] -> Inverter3/1
  Inverter3/1 -> Logical6/2
  Logical4/1 -> Logical2/1
  Inverter4/1 -> Logical4/2
  Logical5/1 -> Logical8/1
  Inverter1/1 -> Logical5/2
  Logical7/1 -> Logical10/1
  Inverter2/1 -> Logical7/2
  [adc_protect_disable] -> Logical5/1
  [adc_protect_disable] -> Logical7/1
  [adc_protect_disable] -> Logical6/1
  Logical6/1 -> Logical3/1
  [adc_protect_disable] -> Logical4/1

== system baopoco/WGNs ==
  Constant : Constant  Value=0
  Constant2 : Constant  Value=0
  Delay1 : xbsIndex_r4/Delay  rst=off  infoeditControl=Selection of Reset will increase slice count due to use of real FFs and instead of SRLs  en=off  latency=3  dbl_ovrd=off  reg_retiming=on  block_type=delay
  Delay2 : xbsIndex_r4/Delay  rst=off  infoeditControl=Selection of Reset will increase slice count due to use of real FFs and instead of SRLs  en=off  latency=3  dbl_ovrd=off  reg_retiming=on  block_type=delay
  Mux : Mux  Inputs=2
  noise0 : Subsystem
  noise1 : Subsystem
  noise3 : Subsystem
  noise4 : Subsystem
  pol_pack0 : Subsystem
  pol_pack1 : Subsystem
  Out1 : Outport  OutDataType=sfix(16)  OutScaling=2^0
  tag scope: n0=global, n1=global
  wires:
  [seed_ld] -> noise1/2(ld)
  [seed_ld] -> noise3/2(ld)
  [seed_ld] -> noise4/2(ld)
  [seed_ld] -> noise0/2(ld)
  [seed1] -> noise1/1(seed)
  [seed2] -> noise3/1(seed)
  [seed3] -> noise4/1(seed)
  [seed0] -> noise0/1(seed)
  Constant2/1 -> Mux/2
  Constant/1 -> Mux/1
  Mux/1 -> Out1/1
  noise0/1(n1) -> pol_pack0/1(In1)
  noise0/2(n2) -> pol_pack0/2(In2)
  noise1/1(n1) -> pol_pack0/3(In3)
  noise1/2(n2) -> pol_pack0/4(In4)
  pol_pack0/1(Out1) -> Delay2/1
  noise3/1(n1) -> pol_pack1/1(In1)
  noise3/2(n2) -> pol_pack1/2(In2)
  noise4/1(n1) -> pol_pack1/3(In3)
  noise4/2(n2) -> pol_pack1/4(In4)
  pol_pack1/1(Out1) -> Delay1/1
  Delay1/1 -> [n1]
  Delay2/1 -> [n0]

== system baopoco/acc_ctrl ==
  mrst : Inport  OutDataType=sfix(16)  OutScaling=2^0
  sync : Inport  Port=2  OutDataType=sfix(16)  OutScaling=2^0
  acc_len : Inport  Port=3  OutDataType=sfix(16)  OutScaling=2^0
  Delay : xbsIndex_r4/Delay  rst=off  infoeditControl=Selection of Reset will increase slice count due to use of real FFs and instead of SRLs  en=off  latency=1  dbl_ovrd=off  reg_retiming=on  block_type=delay
      settings: xl_area=[0, 0, 0, 0, 0, 0, 0]
  Logical : xbsIndex_r4/Logical  logical_function=OR  inputs=2  en=off  latency=0  precision=Full  arith_type=Unsigned  n_bits=8  bin_pt=2  align_bp=on  dbl_ovrd=off  block_type=logical
      settings: xl_area=[0, 0, 0, 0, 0, 0, 0]
  Register : xbsIndex_r4/Register  init=0  rst=on  en=on  dbl_ovrd=off  block_type=register
      settings: xl_area=[0, 0, 0, 0, 0, 0, 0]
  Relational5 : xbsIndex_r4/Relational  mode=a>=b  en=off  latency=0  dbl_ovrd=off  block_type=relational
      settings: xl_area=[0, 0, 0, 0, 0, 0, 0]  block_version=10.1.3
  Shift : xbsIndex_r4/Shift  shift_dir=Left  shift_bits=chan_bits  en=off  latency=0  precision=Full  arith_type="Signed  (2's comp)"  n_bits=8  bin_pt=2  quantization=Truncate  overflow=Wrap  dbl_ovrd=off  block_type=shift
      settings: xl_area=[0, 0, 0, 0, 0, 0, 0]  block_version=10.1.3
  cntr : xbsIndex_r4/Counter  cnt_type=Free Running  cnt_to=Inf  operation=Up  start_count=1  cnt_by_val=1  arith_type=Unsigned  n_bits=32  bin_pt=0  load_pin=off  rst=on  en=off  explicit_period=on  period=1  dbl_ovrd=off  use_behavioral_HDL=off  implementation=Fabric  use_rpm=on  block_type=counter
      settings: xl_area=[0, 0, 0, 0, 0, 0, 0]
  posedge1 : Subsystem  Tag=Rising Edge Detector
  posedge2 : Subsystem  Tag=Rising Edge Detector
  posedge3 : Subsystem  Tag=Rising Edge Detector
  new_acc : Outport  OutDataType=sfix(16)  OutScaling=2^0
  wires:
  sync/1 -> Register/3, Register/1
  mrst/1 -> posedge2/1(In)
  cntr/1 -> Relational5/1
  Shift/1 -> Delay/1
  Delay/1 -> Relational5/2
  posedge1/1(Out) -> Logical/1
  posedge2/1(Out) -> Register/2
  Register/1 -> posedge1/1(In)
  Logical/1 -> cntr/1
  posedge3/1(Out) -> new_acc/1
  acc_len/1 -> Shift/1
  Relational5/1 -> posedge3/1(In), Logical/2

== system baopoco/adc_unpack0 ==
  In1 : Inport
  Reinterpret{,1..3} (4 alike) : xbsIndex_r4/Reinterpret  force_arith_type=on  arith_type="Signed  (2's comp)"  force_bin_pt=on  bin_pt=7  block_type=reinterpret
      settings: infoedit="Changes signal type without altering the binary representation.   You can changed the signal between signed and unsigned, and relocate the binary point.<P><P>Hardware notes: In hardware this block costs nothing.<P><P>Example:  Suppose the input is 6 bits wide, signed, with 2 fractional bits, and the output is forced to unsigned with 0 fractional bits.  Then an input of -2.0 (1110.00 in binary 2's complement) becomes an output of 56 (111000 in binary)."  block_version=10.1.3
  Slice6 : xbsIndex_r4/Slice  nbits=8  boolean_output=off  mode=Lower Bit Location + Width  bit1=0  base1=MSB of Input  bit0=0  base0=LSB of Input  dbl_ovrd=off  block_type=slice
      settings: infoedit="Extracts a given range of bits from each input sample and presents it at the output.  The output type is ordinarily unsigned with binary point at zero, but can be Boolean when the slice is one bit wide.<P><P>Hardware notes: In hardware this block costs nothing."  block_version=10.1.3
  Slice7 : xbsIndex_r4/Slice  nbits=8  boolean_output=off  mode=Lower Bit Location + Width  bit1=0  base1=MSB of Input  bit0=8  base0=LSB of Input  dbl_ovrd=off  block_type=slice
      settings: infoedit="Extracts a given range of bits from each input sample and presents it at the output.  The output type is ordinarily unsigned with binary point at zero, but can be Boolean when the slice is one bit wide.<P><P>Hardware notes: In hardware this block costs nothing."  block_version=10.1.3
  Slice8 : xbsIndex_r4/Slice  nbits=8  boolean_output=off  mode=Lower Bit Location + Width  bit1=0  base1=MSB of Input  bit0=16  base0=LSB of Input  dbl_ovrd=off  block_type=slice
      settings: infoedit="Extracts a given range of bits from each input sample and presents it at the output.  The output type is ordinarily unsigned with binary point at zero, but can be Boolean when the slice is one bit wide.<P><P>Hardware notes: In hardware this block costs nothing."  block_version=10.1.3
  Slice9 : xbsIndex_r4/Slice  nbits=8  boolean_output=off  mode=Upper Bit Location + Width  bit1=0  base1=MSB of Input  bit0=24  base0=LSB of Input  dbl_ovrd=off  block_type=slice
      settings: infoedit="Extracts a given range of bits from each input sample and presents it at the output.  The output type is ordinarily unsigned with binary point at zero, but can be Boolean when the slice is one bit wide.<P><P>Hardware notes: In hardware this block costs nothing."  block_version=10.1.3
  Out1 : Outport
  Out2 : Outport  Port=2
  Out3 : Outport  Port=3
  Out4 : Outport  Port=4
  wires:
  Reinterpret3/1 -> Out4/1
  Reinterpret2/1 -> Out3/1
  Reinterpret1/1 -> Out2/1
  Reinterpret/1 -> Out1/1
  Slice9/1 -> Reinterpret/1
  Slice8/1 -> Reinterpret1/1
  Slice7/1 -> Reinterpret2/1
  Slice6/1 -> Reinterpret3/1
  In1/1 -> Slice9/1, Slice8/1, Slice7/1, Slice6/1

== system baopoco/adc_unpack1 ==
  In1 : Inport
  Reinterpret{,1..3} (4 alike) : xbsIndex_r4/Reinterpret  force_arith_type=on  arith_type="Signed  (2's comp)"  force_bin_pt=on  bin_pt=7  block_type=reinterpret
      settings: infoedit="Changes signal type without altering the binary representation.   You can changed the signal between signed and unsigned, and relocate the binary point.<P><P>Hardware notes: In hardware this block costs nothing.<P><P>Example:  Suppose the input is 6 bits wide, signed, with 2 fractional bits, and the output is forced to unsigned with 0 fractional bits.  Then an input of -2.0 (1110.00 in binary 2's complement) becomes an output of 56 (111000 in binary)."  block_version=10.1.3
  Slice6 : xbsIndex_r4/Slice  nbits=8  boolean_output=off  mode=Lower Bit Location + Width  bit1=0  base1=MSB of Input  bit0=0  base0=LSB of Input  dbl_ovrd=off  block_type=slice
      settings: infoedit="Extracts a given range of bits from each input sample and presents it at the output.  The output type is ordinarily unsigned with binary point at zero, but can be Boolean when the slice is one bit wide.<P><P>Hardware notes: In hardware this block costs nothing."  block_version=10.1.3
  Slice7 : xbsIndex_r4/Slice  nbits=8  boolean_output=off  mode=Lower Bit Location + Width  bit1=0  base1=MSB of Input  bit0=8  base0=LSB of Input  dbl_ovrd=off  block_type=slice
      settings: infoedit="Extracts a given range of bits from each input sample and presents it at the output.  The output type is ordinarily unsigned with binary point at zero, but can be Boolean when the slice is one bit wide.<P><P>Hardware notes: In hardware this block costs nothing."  block_version=10.1.3
  Slice8 : xbsIndex_r4/Slice  nbits=8  boolean_output=off  mode=Lower Bit Location + Width  bit1=0  base1=MSB of Input  bit0=16  base0=LSB of Input  dbl_ovrd=off  block_type=slice
      settings: infoedit="Extracts a given range of bits from each input sample and presents it at the output.  The output type is ordinarily unsigned with binary point at zero, but can be Boolean when the slice is one bit wide.<P><P>Hardware notes: In hardware this block costs nothing."  block_version=10.1.3
  Slice9 : xbsIndex_r4/Slice  nbits=8  boolean_output=off  mode=Upper Bit Location + Width  bit1=0  base1=MSB of Input  bit0=24  base0=LSB of Input  dbl_ovrd=off  block_type=slice
      settings: infoedit="Extracts a given range of bits from each input sample and presents it at the output.  The output type is ordinarily unsigned with binary point at zero, but can be Boolean when the slice is one bit wide.<P><P>Hardware notes: In hardware this block costs nothing."  block_version=10.1.3
  Out1 : Outport
  Out2 : Outport  Port=2
  Out3 : Outport  Port=3
  Out4 : Outport  Port=4
  wires:
  Reinterpret3/1 -> Out4/1
  Reinterpret2/1 -> Out3/1
  Reinterpret1/1 -> Out2/1
  Reinterpret/1 -> Out1/1
  Slice9/1 -> Reinterpret/1
  Slice8/1 -> Reinterpret1/1
  Slice7/1 -> Reinterpret2/1
  Slice6/1 -> Reinterpret3/1
  In1/1 -> Slice9/1, Slice8/1, Slice7/1, Slice6/1
